simulink model "mbr_2048ch_983mhz_real2"  (R2021a) (continued)
== system mbr_2048ch_983mhz_real2 == (continued)
  synth3_2 : Subsystem  Tag=xps:bram  AncestorBlock=xps_library/Memory/shared_bram  mask{arith_type=Unsigned, addr_width=9, data_width=64, reg_prim_output=on, reg_core_output=on, optimization=Minimum_Area, data_bin_pt=0, init_vals=[0:2^9-1], sample_rate=1}
      mask prompts: arith_type "Output Data Type"; addr_width "Address width"; data_width "Data Width"; reg_prim_output "Register Primitive Output"; reg_core_output "Register Core Output"; data_bin_pt "Data Binary Point"; init_vals "Initial values (simulation only)"; sample_rate "Sample rate"
      mask init:
      | shared_bram_mask;
      mask TypeOptions arith_type: Unsigned | Signed  (2's comp)
      mask TypeOptions data_width: 8 | 16 | 32 | 64 | 128
      mask TypeOptions optimization: Minimum_Area | Low_Power
  synth3_3 : Subsystem  Tag=xps:bram  AncestorBlock=xps_library/Memory/shared_bram  mask{arith_type=Unsigned, addr_width=9, data_width=64, reg_prim_output=on, reg_core_output=on, optimization=Minimum_Area, data_bin_pt=0, init_vals=[0:2^9-1], sample_rate=1}
      mask prompts: arith_type "Output Data Type"; addr_width "Address width"; data_width "Data Width"; reg_prim_output "Register Primitive Output"; reg_core_output "Register Core Output"; data_bin_pt "Data Binary Point"; init_vals "Initial values (simulation only)"; sample_rate "Sample rate"
      mask init:
      | shared_bram_mask;
      mask TypeOptions arith_type: Unsigned | Signed  (2's comp)
      mask TypeOptions data_width: 8 | 16 | 32 | 64 | 128
      mask TypeOptions optimization: Minimum_Area | Low_Power
  wires:
  Constant/1 -> adc_snapshot_d/3(trig), adc_snapshot_d/2(we)
  pipeline11/1(q) -> spect_cnt/1
  a2_1/1(data_out) -> Terminator14/1
  power4/1 -> Delay8/1
  fft_wideband_real/2(out00) -> Delay15/1
  Delay10/1 -> bram_mult2_3/1(In1), bram_mult2_2/1(In1), bram_mult2_1/1(In1), bram_mult2_0/1(In1), pipeline162/1(d), Delay34/1
  rfdc/1(m00_axis_tdata) -> munge/1(din), Reinterpret9/1
  fft_wideband_real/6(of) -> Terminator/1
  simple_bram_vacc/1(dout) -> Reinterpret4/1
  a2_0/1(data_out) -> Terminator13/1
  Reinterpret4/1 -> pipeline24/1(d)
  Constant19/1 -> cnt_rst/1(sim_1)
  a2_3/1(data_out) -> Terminator3/1
  Delay15/1 -> Delay5/1
  fft_wideband_real/1(sync_out) -> Delay14/1
  Delay6/1 -> Delay7/1
  fft_wideband_real/3(out01) -> Delay16/1
  fft_wideband_real/4(out02) -> Delay2/1
  Delay14/1 -> Delay10/1
  Delay23/1 -> pipeline3/1(d)
  power3/1 -> Delay4/1
  power2/1 -> Delay26/1
  Constant16/1 -> acc_len/1(sim_1)
  simple_bram_vacc/2(valid) -> pipeline25/1(d), spect_cnt/2
  simple_bram_vacc2/1(dout) -> Reinterpret1/1
  Delay24/1 -> acc_cntrl/2(sync)
  sync_gen/1(sync_out) -> Delay23/1
  pipeline8/1(q) -> [acc_len]
  Delay16/1 -> Delay17/1
  a2_2/1(data_out) -> Terminator2/1
  pipeline9/1(q) -> [cnt_rst]
  Delay34/1 -> Delay24/1
  [cnt_rst] -> acc_cntrl/3(rst)
  Constant2/1 -> fft_wideband_real/2(shift)
  simple_bram_vacc3/1(dout) -> Reinterpret2/1
  pipeline3/1(q) -> fft_wideband_real/1(sync)
  cnt_rst/1(in_reg) -> pipeline9/1(d)
  Delay2/1 -> Delay3/1
  munge/1(dout) -> bus_expand/1(bus_in)
  power1/1 -> Delay25/1
  [acc_len] -> acc_cntrl/1(acc_len)
  acc_len/1(in_reg) -> pipeline8/1(d)
  simple_bram_vacc1/1(dout) -> Reinterpret5/1
  fft_wideband_real/5(out03) -> Delay6/1
  adc_snapshot_d/1(arm_out) -> Terminator4/1
  Reinterpret9/1 -> adc_snapshot_d/1(in_d)
  Reinterpret5/1 -> pipeline16/1(d)
  simple_bram_vacc1/2(valid) -> pipeline17/1(d)
  Reinterpret1/1 -> pipeline18/1(d)
  Reinterpret2/1 -> pipeline21/1(d)
  simple_bram_vacc3/2(valid) -> pipeline23/1(d)
  simple_bram_vacc2/2(valid) -> pipeline19/1(d)
  pipeline5/1(q) -> simple_bram_vacc/1(new_acc)
  pipeline6/1(q) -> simple_bram_vacc/2(din)
  pipeline12/1(q) -> simple_bram_vacc1/1(new_acc)
  pipeline13/1(q) -> simple_bram_vacc1/2(din)
  pipeline22/1(q) -> simple_bram_vacc2/1(new_acc)
  pipeline27/1(q) -> simple_bram_vacc2/2(din)
  pipeline14/1(q) -> simple_bram_vacc3/1(new_acc)
  pipeline15/1(q) -> simple_bram_vacc3/2(din)
  acc_cntrl/1(new_acc) -> pipeline11/1(d), pipeline82/1(d), pipeline90/1(d), pipeline80/1(d), pipeline100/1(d), pipeline59/1(d), pipeline67/1(d), pipeline57/1(d), pipeline77/1(d), pipeline14/1(d), pipeline22/1(d), pipeline12/1(d), pipeline5/1(d)
  pipeline29/1(q) -> pipeline6/1(d)
  pipeline30/1(q) -> pipeline13/1(d)
  pipeline31/1(q) -> pipeline27/1(d)
  pipeline32/1(q) -> pipeline15/1(d)
  Delay25/1 -> pipeline29/1(d)
  Delay26/1 -> pipeline30/1(d)
  Delay4/1 -> pipeline31/1(d)
  Delay8/1 -> pipeline32/1(d)
  Delay33/1 -> pipeline51/1(d)
  Delay29/1 -> pipeline50/1(d)
  Delay22/1 -> pipeline49/1(d)
  Delay21/1 -> pipeline47/1(d)
  pipeline51/1(q) -> pipeline34/1(d)
  pipeline50/1(q) -> pipeline46/1(d)
  pipeline49/1(q) -> pipeline28/1(d)
  pipeline47/1(q) -> pipeline53/1(d)
  acc_cntrl1/1(new_acc) -> pipeline52/1(d), pipeline10/1(d), pipeline41/1(d), pipeline33/1(d), pipeline4/1(d)
  pipeline34/1(q) -> simple_bram_vacc7/2(din)
  pipeline33/1(q) -> simple_bram_vacc7/1(new_acc)
  pipeline46/1(q) -> simple_bram_vacc6/2(din)
  pipeline41/1(q) -> simple_bram_vacc6/1(new_acc)
  pipeline28/1(q) -> simple_bram_vacc5/2(din)
  pipeline10/1(q) -> simple_bram_vacc5/1(new_acc)
  pipeline53/1(q) -> simple_bram_vacc4/2(din)
  pipeline52/1(q) -> simple_bram_vacc4/1(new_acc)
  simple_bram_vacc6/2(valid) -> pipeline38/1(d)
  simple_bram_vacc7/2(valid) -> pipeline42/1(d)
  Reinterpret6/1 -> pipeline40/1(d)
  Reinterpret3/1 -> pipeline37/1(d)
  simple_bram_vacc5/2(valid) -> pipeline36/1(d)
  Reinterpret8/1 -> pipeline35/1(d)
  Reinterpret10/1 -> adc_snapshot_c/1(in_d)
  adc_snapshot_c/1(arm_out) -> Terminator9/1
  fft_wideband_real1/5(out03) -> Delay31/1
  simple_bram_vacc5/1(dout) -> Reinterpret8/1
  Delay27/1 -> power7/1, cmult2/1(a), pipeline155/1(d), bram_mult2_2/2(d)
  power5/1 -> Delay21/1
  munge1/1(dout) -> bus_expand1/1(bus_in)
  Delay18/1 -> Delay27/1
  pipeline48/1(q) -> fft_wideband_real1/1(sync)
  simple_bram_vacc7/1(dout) -> Reinterpret6/1
  Constant3/1 -> fft_wideband_real1/2(shift)
  [cnt_rst] -> acc_cntrl1/3(rst)
  Delay28/1 -> Delay20/1
  b2_2/1(data_out) -> Terminator7/1
  Delay12/1 -> Delay13/1
  sync_gen1/1(sync_out) -> Delay19/1
  Delay20/1 -> acc_cntrl1/2(sync)
  simple_bram_vacc6/1(dout) -> Reinterpret3/1
  simple_bram_vacc4/2(valid) -> spect_cnt1/2, pipeline44/1(d)
  power6/1 -> Delay22/1
  power7/1 -> Delay29/1
  Delay19/1 -> pipeline48/1(d)
  Delay9/1 -> Delay1/1
  fft_wideband_real1/4(out02) -> Delay18/1
  fft_wideband_real1/3(out01) -> Delay12/1
  Delay31/1 -> Delay32/1
  fft_wideband_real1/1(sync_out) -> Delay9/1
  Delay11/1 -> Delay30/1
  Delay13/1 -> power6/1, cmult1/1(a), pipeline154/1(d), bram_mult2_1/2(d)
  b2_3/1(data_out) -> Terminator8/1
  Reinterpret7/1 -> pipeline43/1(d)
  b2_0/1(data_out) -> Terminator5/1
  spect_cnt1/1 -> pipeline45/1(d), pipeline39/1(d), pipeline54/1(d), pipeline2/1(d)
  simple_bram_vacc4/1(dout) -> Reinterpret7/1
  Delay32/1 -> power8/1, cmult3/1(a), pipeline156/1(d), bram_mult2_3/2(d)
  Delay30/1 -> cmult/1(a), pipeline153/1(d), bram_mult2_0/2(d), power5/1
  fft_wideband_real1/6(of) -> Terminator1/1
  Delay1/1 -> pipeline164/1(d), bram_mult3_0/1(In1), bram_mult3_1/1(In1), bram_mult3_2/1(In1), bram_mult3_3/1(In1), Delay28/1
  fft_wideband_real1/2(out00) -> Delay11/1
  power8/1 -> Delay33/1
  b2_1/1(data_out) -> Terminator6/1
  pipeline4/1(q) -> spect_cnt1/1
  Constant1/1 -> adc_snapshot_c/2(we), adc_snapshot_c/3(trig)
  rfdc/2(m02_axis_tdata) -> Reinterpret10/1, munge1/1(din)
  [acc_len] -> acc_cntrl1/1(acc_len)
  rfdc/3(m10_axis_tdata) -> Terminator10/1
  rfdc/4(m12_axis_tdata) -> Terminator11/1
  rfdc/8(m32_axis_tdata) -> Terminator17/1
  rfdc/7(m30_axis_tdata) -> Terminator16/1
  Band-Limited White Noise/1 -> rfdc/8(m32_axis_tdata_sim), rfdc/7(m30_axis_tdata_sim), rfdc/6(m22_axis_tdata_sim), rfdc/5(m20_axis_tdata_sim), rfdc/4(m12_axis_tdata_sim), rfdc/3(m10_axis_tdata_sim), rfdc/2(m02_axis_tdata_sim), rfdc/1(m00_axis_tdata_sim)
  Delay40/1 -> pipeline76/1(d)
  Delay39/1 -> pipeline75/1(d)
  Delay37/1 -> pipeline74/1(d)
  Delay36/1 -> pipeline73/1(d)
  pipeline76/1(q) -> pipeline60/1(d)
  pipeline75/1(q) -> pipeline72/1(d)
  pipeline74/1(q) -> pipeline58/1(d)
  pipeline73/1(q) -> pipeline78/1(d)
  pipeline60/1(q) -> simple_bram_vacc11/2(din)
  pipeline59/1(q) -> simple_bram_vacc11/1(new_acc)
  pipeline72/1(q) -> simple_bram_vacc10/2(din)
  pipeline67/1(q) -> simple_bram_vacc10/1(new_acc)
  pipeline58/1(q) -> simple_bram_vacc9/2(din)
  pipeline57/1(q) -> simple_bram_vacc9/1(new_acc)
  pipeline78/1(q) -> simple_bram_vacc8/2(din)
  pipeline77/1(q) -> simple_bram_vacc8/1(new_acc)
  simple_bram_vacc10/2(valid) -> pipeline64/1(d)
  simple_bram_vacc11/2(valid) -> pipeline68/1(d)
  Reinterpret12/1 -> pipeline66/1(d)
  Reinterpret11/1 -> pipeline63/1(d)
  simple_bram_vacc9/2(valid) -> pipeline62/1(d)
  Reinterpret14/1 -> pipeline61/1(d)
  simple_bram_vacc9/1(dout) -> Reinterpret14/1
  c_to_ri/1 -> Delay36/1
  simple_bram_vacc11/1(dout) -> Reinterpret12/1
  ab_re1/1(data_out) -> Terminator20/1
  simple_bram_vacc10/1(dout) -> Reinterpret11/1
  simple_bram_vacc8/2(valid) -> pipeline70/1(d)
  c_to_ri/2 -> Delay37/1
  c_to_ri1/1 -> Delay39/1
  ab_im1/1(data_out) -> Terminator21/1
  Reinterpret13/1 -> pipeline69/1(d)
  ab_re0/1(data_out) -> Terminator18/1
  spect_cnt/1 -> pipeline1/1(d), pipeline7/1(d), pipeline20/1(d), pipeline56/1(d), pipeline94/1(d), pipeline88/1(d), pipeline102/1(d), pipeline71/1(d), pipeline65/1(d), pipeline79/1(d), pipeline55/1(d), pipeline26/1(d)
  simple_bram_vacc8/1(dout) -> Reinterpret13/1
  c_to_ri1/2 -> Delay40/1
  ab_im0/1(data_out) -> Terminator19/1
  ab_im2/1(data_out) -> Terminator23/1
  c_to_ri3/2 -> Delay42/1
  simple_bram_vacc14/1(dout) -> Reinterpret17/1
  ab_re2/1(data_out) -> Terminator22/1
  Reinterpret17/1 -> pipeline92/1(d)
  ab_im3/1(data_out) -> Terminator25/1
  c_to_ri3/1 -> Delay41/1
  c_to_ri2/2 -> Delay38/1
  simple_bram_vacc14/2(valid) -> pipeline93/1(d)
  simple_bram_vacc12/1(dout) -> Reinterpret15/1
  ab_re3/1(data_out) -> Terminator24/1
  simple_bram_vacc13/1(dout) -> Reinterpret16/1
  c_to_ri2/1 -> Delay35/1
  simple_bram_vacc15/1(dout) -> Reinterpret18/1
  Reinterpret18/1 -> pipeline84/1(d)
  simple_bram_vacc15/2(valid) -> pipeline85/1(d)
  Reinterpret15/1 -> pipeline86/1(d)
  Reinterpret16/1 -> pipeline89/1(d)
  simple_bram_vacc13/2(valid) -> pipeline91/1(d)
  simple_bram_vacc12/2(valid) -> pipeline87/1(d)
  pipeline100/1(q) -> simple_bram_vacc14/1(new_acc)
  pipeline101/1(q) -> simple_bram_vacc14/2(din)
  pipeline80/1(q) -> simple_bram_vacc15/1(new_acc)
  pipeline81/1(q) -> simple_bram_vacc15/2(din)
  pipeline90/1(q) -> simple_bram_vacc12/1(new_acc)
  pipeline95/1(q) -> simple_bram_vacc12/2(din)
  pipeline82/1(q) -> simple_bram_vacc13/1(new_acc)
  pipeline83/1(q) -> simple_bram_vacc13/2(din)
  pipeline96/1(q) -> pipeline101/1(d)
  pipeline97/1(q) -> pipeline81/1(d)
  pipeline98/1(q) -> pipeline95/1(d)
  pipeline99/1(q) -> pipeline83/1(d)
  Delay35/1 -> pipeline96/1(d)
  Delay38/1 -> pipeline97/1(d)
  Delay41/1 -> pipeline98/1(d)
  Delay42/1 -> pipeline99/1(d)
  cmult/1(ab) -> c_to_ri/1
  cmult1/1(ab) -> c_to_ri1/1
  cmult3/1(ab) -> c_to_ri3/1
  cmult2/1(ab) -> c_to_ri2/1
  [acc_len] -> acc_cntrl2/1(acc_len)
  pipeline113/1(q) -> spect_cnt2/1
  synth1_1/1(data_out) -> Terminator27/1
  power12/1 -> Delay48/1
  simple_bram_vacc16/1(dout) -> Reinterpret21/1
  spect_cnt2/1 -> pipeline104/1(d), pipeline127/1(d), pipeline112/1(d), pipeline119/1(d)
  synth1_0/1(data_out) -> Terminator26/1
  Reinterpret21/1 -> pipeline117/1(d)
  synth1_3/1(data_out) -> Terminator29/1
  power11/1 -> Delay47/1
  power10/1 -> Delay45/1
  simple_bram_vacc16/2(valid) -> pipeline118/1(d), spect_cnt2/2
  simple_bram_vacc18/1(dout) -> Reinterpret19/1
  Delay43/1 -> acc_cntrl2/2(sync)
  synth1_2/1(data_out) -> Terminator28/1
  Delay46/1 -> Delay43/1
  [cnt_rst] -> acc_cntrl2/3(rst)
  simple_bram_vacc19/1(dout) -> Reinterpret20/1
  power9/1 -> Delay44/1
  simple_bram_vacc17/1(dout) -> Reinterpret22/1
  Reinterpret22/1 -> pipeline108/1(d)
  simple_bram_vacc17/2(valid) -> pipeline109/1(d)
  Reinterpret19/1 -> pipeline110/1(d)
  Reinterpret20/1 -> pipeline114/1(d)
  simple_bram_vacc19/2(valid) -> pipeline116/1(d)
  simple_bram_vacc18/2(valid) -> pipeline111/1(d)
  pipeline125/1(q) -> simple_bram_vacc16/1(new_acc)
  pipeline126/1(q) -> simple_bram_vacc16/2(din)
  pipeline103/1(q) -> simple_bram_vacc17/1(new_acc)
  pipeline105/1(q) -> simple_bram_vacc17/2(din)
  pipeline115/1(q) -> simple_bram_vacc18/1(new_acc)
  pipeline120/1(q) -> simple_bram_vacc18/2(din)
  pipeline106/1(q) -> simple_bram_vacc19/1(new_acc)
  pipeline107/1(q) -> simple_bram_vacc19/2(din)
  acc_cntrl2/1(new_acc) -> pipeline113/1(d), pipeline106/1(d), pipeline115/1(d), pipeline103/1(d), pipeline125/1(d)
  pipeline121/1(q) -> pipeline126/1(d)
  pipeline122/1(q) -> pipeline105/1(d)
  pipeline123/1(q) -> pipeline120/1(d)
  pipeline124/1(q) -> pipeline107/1(d)
  Delay44/1 -> pipeline121/1(d)
  Delay45/1 -> pipeline122/1(d)
  Delay47/1 -> pipeline123/1(d)
  Delay48/1 -> pipeline124/1(d)
  Delay54/1 -> pipeline149/1(d)
  Delay53/1 -> pipeline148/1(d)
  Delay51/1 -> pipeline147/1(d)
  Delay50/1 -> pipeline146/1(d)
  pipeline149/1(q) -> pipeline132/1(d)
  pipeline148/1(q) -> pipeline145/1(d)
  pipeline147/1(q) -> pipeline130/1(d)
  pipeline146/1(q) -> pipeline151/1(d)
  acc_cntrl3/1(new_acc) -> pipeline150/1(d), pipeline128/1(d), pipeline140/1(d), pipeline131/1(d), pipeline138/1(d)
  pipeline132/1(q) -> simple_bram_vacc23/2(din)
  pipeline131/1(q) -> simple_bram_vacc23/1(new_acc)
  pipeline145/1(q) -> simple_bram_vacc22/2(din)
  pipeline140/1(q) -> simple_bram_vacc22/1(new_acc)
  pipeline130/1(q) -> simple_bram_vacc21/2(din)
  pipeline128/1(q) -> simple_bram_vacc21/1(new_acc)
  pipeline151/1(q) -> simple_bram_vacc20/2(din)
  pipeline150/1(q) -> simple_bram_vacc20/1(new_acc)
  simple_bram_vacc22/2(valid) -> pipeline136/1(d)
  simple_bram_vacc23/2(valid) -> pipeline141/1(d)
  Reinterpret24/1 -> pipeline139/1(d)
  Reinterpret23/1 -> pipeline135/1(d)
  simple_bram_vacc21/2(valid) -> pipeline134/1(d)
  Reinterpret26/1 -> pipeline133/1(d)
  simple_bram_vacc21/1(dout) -> Reinterpret26/1
  power16/1 -> Delay50/1
  simple_bram_vacc23/1(dout) -> Reinterpret24/1
  [cnt_rst] -> acc_cntrl3/3(rst)
  Delay52/1 -> Delay49/1
  synth0_2/1(data_out) -> Terminator32/1
  Delay49/1 -> acc_cntrl3/2(sync)
  simple_bram_vacc22/1(dout) -> Reinterpret23/1
  simple_bram_vacc20/2(valid) -> spect_cnt3/2, pipeline143/1(d)
  power13/1 -> Delay51/1
  power14/1 -> Delay53/1
  synth0_3/1(data_out) -> Terminator33/1
  Reinterpret25/1 -> pipeline142/1(d)
  synth0_0/1(data_out) -> Terminator30/1
  spect_cnt3/1 -> pipeline144/1(d), pipeline137/1(d), pipeline152/1(d), pipeline129/1(d)
  simple_bram_vacc20/1(dout) -> Reinterpret25/1
  power15/1 -> Delay54/1
  synth0_1/1(data_out) -> Terminator31/1
  pipeline138/1(q) -> spect_cnt3/1
  [acc_len] -> acc_cntrl3/1(acc_len)
  comp_add4/1(a+b) -> power16/1
  comp_add5/1(a+b) -> power13/1
  comp_add6/1(a+b) -> power14/1
  comp_add7/1(a+b) -> power15/1
  bram_mult3_0/1(c) -> comp_add4/2(b)
  pipeline153/1(q) -> comp_add4/1(a)
  bram_mult3_1/1(c) -> comp_add5/2(b)
  pipeline154/1(q) -> comp_add5/1(a)
  pipeline156/1(q) -> comp_add7/1(a)
  bram_mult3_3/1(c) -> comp_add7/2(b)
  pipeline155/1(q) -> comp_add6/1(a)
  bram_mult3_2/1(c) -> comp_add6/2(b)
  bram_mult2_2/1(c) -> comp_add2/2(b)
  pipeline159/1(q) -> comp_add2/1(a)
  bram_mult2_3/1(c) -> comp_add3/2(b)
  pipeline160/1(q) -> comp_add3/1(a)
  pipeline158/1(q) -> comp_add1/1(a)
  bram_mult2_1/1(c) -> comp_add1/2(b)
  pipeline157/1(q) -> comp_add/1(a)
  bram_mult2_0/1(c) -> comp_add/2(b)
  comp_add3/1(a+b) -> Delay112/1, power12/1
  comp_add2/1(a+b) -> Delay111/1, power11/1
  comp_add1/1(a+b) -> Delay110/1, power10/1
  comp_add/1(a+b) -> Delay109/1, power9/1
  pipeline161/1(q) -> Delay46/1, Delay113/1
  pipeline162/1(q) -> pipeline161/1(d)
  pipeline164/1(q) -> pipeline163/1(d)
  pipeline163/1(q) -> Delay52/1
  Delay5/1 -> cmult/2(b), bram_mult3_0/2(d), pipeline157/1(d), power1/1
  Delay17/1 -> power2/1, cmult1/2(b), bram_mult3_1/2(d), pipeline158/1(d)
  Delay3/1 -> power3/1, cmult2/2(b), bram_mult3_2/2(d), pipeline159/1(d)
  Delay7/1 -> power4/1, cmult3/2(b), bram_mult3_3/2(d), pipeline160/1(d)
  Delay106/1 -> power27/1, bram_mult1_3/2(d), pipeline228/1(d), cmult7/2(b)
  Delay77/1 -> bram_mult1_2/2(d), pipeline227/1(d), power26/1, cmult6/2(b)
  Delay63/1 -> cmult5/2(b), power25/1, bram_mult1_1/2(d), pipeline226/1(d)
  Delay99/1 -> bram_mult1_0/2(d), pipeline225/1(d), cmult4/2(b), power17/1
  pipeline236/1(q) -> Delay102/1
  pipeline237/1(q) -> pipeline236/1(d)
  pipeline235/1(q) -> pipeline234/1(d)
  pipeline234/1(q) -> inverter4/1(sync), inverter1/1(sync), inverter2/1(sync), inverter3/1(sync), Delay95/1
  comp_add8/1(a+b) -> inverter3/2(din), power32/1
  comp_add9/1(a+b) -> inverter2/2(din), power18/1
  comp_add10/1(a+b) -> inverter1/2(din), power19/1
  comp_add11/1(a+b) -> inverter4/2(din), power20/1
  bram_mult1_0/1(c) -> comp_add8/2(b)
  pipeline229/1(q) -> comp_add8/1(a)
  bram_mult1_1/1(c) -> comp_add9/2(b)
  pipeline230/1(q) -> comp_add9/1(a)
  pipeline233/1(q) -> comp_add11/1(a)
  bram_mult1_3/1(c) -> comp_add11/2(b)
  pipeline231/1(q) -> comp_add10/1(a)
  bram_mult1_2/1(c) -> comp_add10/2(b)
  bram_mult0_2/1(c) -> comp_add14/2(b)
  pipeline227/1(q) -> comp_add14/1(a)
  bram_mult0_3/1(c) -> comp_add15/2(b)
  pipeline228/1(q) -> comp_add15/1(a)
  pipeline226/1(q) -> comp_add13/1(a)
  bram_mult0_1/1(c) -> comp_add13/2(b)
  pipeline225/1(q) -> comp_add12/1(a)
  bram_mult0_0/1(c) -> comp_add12/2(b)
  comp_add15/1(a+b) -> power23/1
  comp_add14/1(a+b) -> power22/1
  comp_add13/1(a+b) -> power21/1
  comp_add12/1(a+b) -> power24/1
  [acc_len] -> acc_cntrl7/1(acc_len)
  pipeline208/1(q) -> spect_cnt7/1
  synth3_1/1(data_out) -> Terminator53/1
  power23/1 -> Delay104/1
  simple_bram_vacc37/1(dout) -> Reinterpret44/1
  spect_cnt7/1 -> pipeline198/1(d), pipeline224/1(d), pipeline207/1(d), pipeline215/1(d)
  synth3_0/1(data_out) -> Terminator52/1
  Reinterpret44/1 -> pipeline213/1(d)
  synth3_3/1(data_out) -> Terminator55/1
  power22/1 -> Delay103/1
  power21/1 -> Delay101/1
  simple_bram_vacc37/2(valid) -> pipeline214/1(d), spect_cnt7/2
  simple_bram_vacc39/1(dout) -> Reinterpret42/1
  Delay98/1 -> acc_cntrl7/2(sync)
  synth3_2/1(data_out) -> Terminator54/1
  Delay102/1 -> Delay98/1
  [cnt_rst] -> acc_cntrl7/3(rst)
  simple_bram_vacc40/1(dout) -> Reinterpret43/1
  power24/1 -> Delay100/1
  simple_bram_vacc38/1(dout) -> Reinterpret45/1
  Reinterpret45/1 -> pipeline203/1(d)
  simple_bram_vacc38/2(valid) -> pipeline204/1(d)
  Reinterpret42/1 -> pipeline205/1(d)
  Reinterpret43/1 -> pipeline209/1(d)
  simple_bram_vacc40/2(valid) -> pipeline212/1(d)
  simple_bram_vacc39/2(valid) -> pipeline206/1(d)
  pipeline222/1(q) -> simple_bram_vacc37/1(new_acc)
  pipeline223/1(q) -> simple_bram_vacc37/2(din)
  pipeline197/1(q) -> simple_bram_vacc38/1(new_acc)
  pipeline200/1(q) -> simple_bram_vacc38/2(din)
  pipeline211/1(q) -> simple_bram_vacc39/1(new_acc)
  pipeline216/1(q) -> simple_bram_vacc39/2(din)
  pipeline201/1(q) -> simple_bram_vacc40/1(new_acc)
  pipeline202/1(q) -> simple_bram_vacc40/2(din)
  acc_cntrl7/1(new_acc) -> pipeline208/1(d), pipeline201/1(d), pipeline211/1(d), pipeline197/1(d), pipeline222/1(d)
  pipeline217/1(q) -> pipeline223/1(d)
  pipeline218/1(q) -> pipeline200/1(d)
  pipeline219/1(q) -> pipeline216/1(d)
  pipeline220/1(q) -> pipeline202/1(d)
  Delay100/1 -> pipeline217/1(d)
  Delay101/1 -> pipeline218/1(d)
  Delay103/1 -> pipeline219/1(d)
  Delay104/1 -> pipeline220/1(d)
  Delay97/1 -> pipeline193/1(d)
  Delay96/1 -> pipeline192/1(d)
  Delay94/1 -> pipeline191/1(d)
  Delay93/1 -> pipeline190/1(d)
  pipeline193/1(q) -> pipeline174/1(d)
  pipeline192/1(q) -> pipeline189/1(d)
  pipeline191/1(q) -> pipeline172/1(d)
  pipeline190/1(q) -> pipeline195/1(d)
  acc_cntrl6/1(new_acc) -> pipeline194/1(d), pipeline170/1(d), pipeline183/1(d), pipeline173/1(d), pipeline181/1(d)
  pipeline174/1(q) -> simple_bram_vacc35/2(din)
  pipeline173/1(q) -> simple_bram_vacc35/1(new_acc)
  pipeline189/1(q) -> simple_bram_vacc34/2(din)
  pipeline183/1(q) -> simple_bram_vacc34/1(new_acc)
  pipeline172/1(q) -> simple_bram_vacc33/2(din)
  pipeline170/1(q) -> simple_bram_vacc33/1(new_acc)
  pipeline195/1(q) -> simple_bram_vacc32/2(din)
  pipeline194/1(q) -> simple_bram_vacc32/1(new_acc)
  simple_bram_vacc34/2(valid) -> pipeline179/1(d)
  simple_bram_vacc35/2(valid) -> pipeline184/1(d)
  Reinterpret39/1 -> pipeline182/1(d)
  Reinterpret37/1 -> pipeline178/1(d)
  simple_bram_vacc33/2(valid) -> pipeline176/1(d)
  Reinterpret41/1 -> pipeline175/1(d)
  simple_bram_vacc33/1(dout) -> Reinterpret41/1
  power32/1 -> Delay93/1
  simple_bram_vacc35/1(dout) -> Reinterpret39/1
  [cnt_rst] -> acc_cntrl6/3(rst)
  Delay95/1 -> Delay92/1
  synth2_2/1(data_out) -> Terminator49/1
  Delay92/1 -> acc_cntrl6/2(sync)
  simple_bram_vacc34/1(dout) -> Reinterpret37/1
  simple_bram_vacc32/2(valid) -> spect_cnt6/2, pipeline186/1(d)
  power18/1 -> Delay94/1
  power19/1 -> Delay96/1
  synth2_3/1(data_out) -> Terminator50/1
  Reinterpret40/1 -> pipeline185/1(d)
  synth2_0/1(data_out) -> Terminator47/1
  spect_cnt6/1 -> pipeline187/1(d), pipeline180/1(d), pipeline196/1(d), pipeline171/1(d)
  simple_bram_vacc32/1(dout) -> Reinterpret40/1
  power20/1 -> Delay97/1
  synth2_1/1(data_out) -> Terminator48/1
  pipeline181/1(q) -> spect_cnt6/1
  [acc_len] -> acc_cntrl6/1(acc_len)
  cmult6/1(ab) -> c_to_ri6/1
  cmult7/1(ab) -> c_to_ri7/1
  cmult5/1(ab) -> c_to_ri5/1
  cmult4/1(ab) -> c_to_ri4/1
  Delay91/1 -> pipeline326/1(d)
  Delay90/1 -> pipeline325/1(d)
  Delay86/1 -> pipeline324/1(d)
  Delay83/1 -> pipeline323/1(d)
  pipeline326/1(q) -> pipeline310/1(d)
  pipeline325/1(q) -> pipeline322/1(d)
  pipeline324/1(q) -> pipeline308/1(d)
  pipeline323/1(q) -> pipeline168/1(d)
  pipeline310/1(q) -> simple_bram_vacc29/2(din)
  pipeline309/1(q) -> simple_bram_vacc29/1(new_acc)
  pipeline322/1(q) -> simple_bram_vacc28/2(din)
  pipeline317/1(q) -> simple_bram_vacc28/1(new_acc)
  pipeline308/1(q) -> simple_bram_vacc31/2(din)
  pipeline307/1(q) -> simple_bram_vacc31/1(new_acc)
  pipeline168/1(q) -> simple_bram_vacc30/2(din)
  pipeline167/1(q) -> simple_bram_vacc30/1(new_acc)
  simple_bram_vacc28/2(valid) -> pipeline314/1(d)
  simple_bram_vacc29/2(valid) -> pipeline318/1(d)
  Reinterpret34/1 -> pipeline316/1(d)
  Reinterpret33/1 -> pipeline313/1(d)
  simple_bram_vacc31/2(valid) -> pipeline312/1(d)
  Reinterpret36/1 -> pipeline311/1(d)
  simple_bram_vacc31/1(dout) -> Reinterpret36/1
  c_to_ri6/1 -> Delay83/1
  simple_bram_vacc29/1(dout) -> Reinterpret34/1
  cd_re3/1(data_out) -> Terminator45/1
  simple_bram_vacc28/1(dout) -> Reinterpret33/1
  simple_bram_vacc30/2(valid) -> pipeline320/1(d)
  c_to_ri6/2 -> Delay86/1
  c_to_ri7/1 -> Delay90/1
  cd_im3/1(data_out) -> Terminator46/1
  Reinterpret35/1 -> pipeline319/1(d)
  cd_re2/1(data_out) -> Terminator43/1
  simple_bram_vacc30/1(dout) -> Reinterpret35/1
  c_to_ri7/2 -> Delay91/1
  cd_im2/1(data_out) -> Terminator44/1
  cd_im0/1(data_out) -> Terminator39/1
  c_to_ri5/2 -> Delay89/1
  simple_bram_vacc46/1(dout) -> Reinterpret31/1
  spect_cnt4/1 -> pipeline248/1(d), pipeline280/1(d), pipeline306/1(d), pipeline291/1(d), pipeline298/1(d), pipeline169/1(d), pipeline315/1(d), pipeline321/1(d), pipeline281/1(d), pipeline242/1(d), pipeline296/1(d), pipeline165/1(d)
  cd_re0/1(data_out) -> Terminator38/1
  Reinterpret31/1 -> pipeline295/1(d)
  cd_im1/1(data_out) -> Terminator42/1
  c_to_ri5/1 -> Delay87/1
  c_to_ri4/2 -> Delay85/1
  simple_bram_vacc46/2(valid) -> pipeline297/1(d)
  simple_bram_vacc26/1(dout) -> Reinterpret29/1
  cd_re1/1(data_out) -> Terminator41/1
  simple_bram_vacc27/1(dout) -> Reinterpret30/1
  c_to_ri4/1 -> Delay84/1
  simple_bram_vacc47/1(dout) -> Reinterpret32/1
  Reinterpret32/1 -> pipeline287/1(d)
  simple_bram_vacc47/2(valid) -> pipeline288/1(d)
  Reinterpret29/1 -> pipeline289/1(d)
  Reinterpret30/1 -> pipeline292/1(d)
  simple_bram_vacc27/2(valid) -> pipeline294/1(d)
  simple_bram_vacc26/2(valid) -> pipeline290/1(d)
  pipeline304/1(q) -> simple_bram_vacc46/1(new_acc)
  pipeline305/1(q) -> simple_bram_vacc46/2(din)
  pipeline282/1(q) -> simple_bram_vacc47/1(new_acc)
  pipeline283/1(q) -> simple_bram_vacc47/2(din)
  pipeline293/1(q) -> simple_bram_vacc26/1(new_acc)
  pipeline299/1(q) -> simple_bram_vacc26/2(din)
  pipeline284/1(q) -> simple_bram_vacc27/1(new_acc)
  pipeline286/1(q) -> simple_bram_vacc27/2(din)
  pipeline300/1(q) -> pipeline305/1(d)
  pipeline301/1(q) -> pipeline283/1(d)
  pipeline302/1(q) -> pipeline299/1(d)
  pipeline303/1(q) -> pipeline286/1(d)
  Delay84/1 -> pipeline300/1(d)
  Delay85/1 -> pipeline301/1(d)
  Delay87/1 -> pipeline302/1(d)
  Delay89/1 -> pipeline303/1(d)
  [acc_len] -> acc_cntrl5/1(acc_len)
  rfdc/6(m22_axis_tdata) -> Reinterpret28/1, munge3/1(din)
  Constant5/1 -> adc_snapshot_a/3(trig), adc_snapshot_a/2(we)
  pipeline263/1(q) -> spect_cnt5/1
  d2_1/1(data_out) -> Terminator58/1
  power31/1 -> Delay81/1
  fft_wideband_real3/2(out00) -> Delay57/1
  Delay55/1 -> bram_mult1_0/1(In1), bram_mult1_3/1(In1), bram_mult1_2/1(In1), bram_mult1_1/1(In1), pipeline235/1(d), Delay75/1
  fft_wideband_real3/6(of) -> Terminator35/1
  Delay78/1 -> power28/1, cmult4/1(a), bram_mult0_0/2(d), pipeline229/1(d)
  Delay80/1 -> power31/1, cmult7/1(a), bram_mult0_3/2(d), pipeline233/1(d)
  simple_bram_vacc42/1(dout) -> Reinterpret50/1
  spect_cnt5/1 -> pipeline241/1(d), pipeline279/1(d), pipeline262/1(d), pipeline269/1(d)
  d2_0/1(data_out) -> Terminator57/1
  Reinterpret50/1 -> pipeline267/1(d)
  d2_3/1(data_out) -> Terminator60/1
  Delay57/1 -> Delay78/1
  fft_wideband_real3/1(sync_out) -> Delay108/1
  Delay79/1 -> Delay80/1
  fft_wideband_real3/3(out01) -> Delay58/1
  fft_wideband_real3/4(out02) -> Delay64/1
  Delay108/1 -> Delay55/1
  Delay65/1 -> pipeline272/1(d)
  power30/1 -> Delay76/1
  power29/1 -> Delay69/1
  simple_bram_vacc42/2(valid) -> pipeline268/1(d), spect_cnt5/2
  simple_bram_vacc44/1(dout) -> Reinterpret46/1
  Delay67/1 -> acc_cntrl5/2(sync)
  sync_gen3/1(sync_out) -> Delay65/1
  Delay58/1 -> Delay59/1
  d2_2/1(data_out) -> Terminator59/1
  Delay75/1 -> Delay67/1
  [cnt_rst] -> acc_cntrl5/3(rst)
  Constant7/1 -> fft_wideband_real3/2(shift)
  simple_bram_vacc45/1(dout) -> Reinterpret49/1
  pipeline272/1(q) -> fft_wideband_real3/1(sync)
  Delay64/1 -> Delay74/1
  munge3/1(dout) -> bus_expand3/1(bus_in)
  power28/1 -> Delay68/1
  simple_bram_vacc43/1(dout) -> Reinterpret51/1
  fft_wideband_real3/5(out03) -> Delay79/1
  adc_snapshot_a/1(arm_out) -> Terminator61/1
  Reinterpret28/1 -> adc_snapshot_a/1(in_d)
  Reinterpret51/1 -> pipeline258/1(d)
  simple_bram_vacc43/2(valid) -> pipeline259/1(d)
  Reinterpret46/1 -> pipeline260/1(d)
  Reinterpret49/1 -> pipeline264/1(d)
  simple_bram_vacc45/2(valid) -> pipeline266/1(d)
  simple_bram_vacc44/2(valid) -> pipeline261/1(d)
  pipeline277/1(q) -> simple_bram_vacc42/1(new_acc)
  pipeline278/1(q) -> simple_bram_vacc42/2(din)
  pipeline166/1(q) -> simple_bram_vacc43/1(new_acc)
  pipeline250/1(q) -> simple_bram_vacc43/2(din)
  pipeline265/1(q) -> simple_bram_vacc44/1(new_acc)
  pipeline270/1(q) -> simple_bram_vacc44/2(din)
  pipeline256/1(q) -> simple_bram_vacc45/1(new_acc)
  pipeline257/1(q) -> simple_bram_vacc45/2(din)
  acc_cntrl5/1(new_acc) -> pipeline263/1(d), pipeline256/1(d), pipeline265/1(d), pipeline166/1(d), pipeline277/1(d)
  pipeline271/1(q) -> pipeline278/1(d)
  pipeline273/1(q) -> pipeline250/1(d)
  pipeline275/1(q) -> pipeline270/1(d)
  pipeline276/1(q) -> pipeline257/1(d)
  Delay68/1 -> pipeline271/1(d)
  Delay69/1 -> pipeline273/1(d)
  Delay76/1 -> pipeline275/1(d)
  Delay81/1 -> pipeline276/1(d)
  Delay107/1 -> pipeline255/1(d)
  Delay88/1 -> pipeline254/1(d)
  Delay73/1 -> pipeline253/1(d)
  Delay72/1 -> pipeline251/1(d)
  pipeline255/1(q) -> pipeline221/1(d)
  pipeline254/1(q) -> pipeline249/1(d)
  pipeline253/1(q) -> pipeline199/1(d)
  pipeline251/1(q) -> pipeline285/1(d)
  acc_cntrl4/1(new_acc) -> pipeline274/1(d), pipeline188/1(d), pipeline244/1(d), pipeline210/1(d), pipeline304/1(d), pipeline282/1(d), pipeline293/1(d), pipeline284/1(d), pipeline167/1(d), pipeline307/1(d), pipeline317/1(d), pipeline309/1(d), pipeline177/1(d)
  pipeline221/1(q) -> simple_bram_vacc41/2(din)
  pipeline210/1(q) -> simple_bram_vacc41/1(new_acc)
  pipeline249/1(q) -> simple_bram_vacc36/2(din)
  pipeline244/1(q) -> simple_bram_vacc36/1(new_acc)
  pipeline199/1(q) -> simple_bram_vacc25/2(din)
  pipeline188/1(q) -> simple_bram_vacc25/1(new_acc)
  pipeline285/1(q) -> simple_bram_vacc24/2(din)
  pipeline274/1(q) -> simple_bram_vacc24/1(new_acc)
  simple_bram_vacc36/2(valid) -> pipeline240/1(d)
  simple_bram_vacc41/2(valid) -> pipeline245/1(d)
  Reinterpret38/1 -> pipeline243/1(d)
  Reinterpret27/1 -> pipeline239/1(d)
  simple_bram_vacc25/2(valid) -> pipeline238/1(d)
  Reinterpret48/1 -> pipeline232/1(d)
  Reinterpret52/1 -> adc_snapshot_b/1(in_d)
  adc_snapshot_b/1(arm_out) -> Terminator56/1
  fft_wideband_real2/5(out03) -> Delay105/1
  simple_bram_vacc25/1(dout) -> Reinterpret48/1
  [acc_len] -> acc_cntrl4/1(acc_len)
  power17/1 -> Delay72/1
  munge2/1(dout) -> bus_expand2/1(bus_in)
  Delay66/1 -> Delay77/1
  pipeline252/1(q) -> fft_wideband_real2/1(sync)
  simple_bram_vacc41/1(dout) -> Reinterpret38/1
  Constant6/1 -> fft_wideband_real2/2(shift)
  [cnt_rst] -> acc_cntrl4/3(rst)
  Delay82/1 -> Delay71/1
  c2_2/1(data_out) -> Terminator40/1
  Delay62/1 -> Delay63/1
  sync_gen2/1(sync_out) -> Delay70/1
  Delay71/1 -> acc_cntrl4/2(sync)
  simple_bram_vacc36/1(dout) -> Reinterpret27/1
  simple_bram_vacc24/2(valid) -> spect_cnt4/2, pipeline247/1(d)
  power25/1 -> Delay73/1
  power26/1 -> Delay88/1
  Delay70/1 -> pipeline252/1(d)
  Delay60/1 -> Delay56/1
  fft_wideband_real2/4(out02) -> Delay66/1
  fft_wideband_real2/3(out01) -> Delay62/1
  Delay105/1 -> Delay106/1
  fft_wideband_real2/1(sync_out) -> Delay60/1
  Delay61/1 -> Delay99/1
  c2_3/1(data_out) -> Terminator51/1
  Reinterpret47/1 -> pipeline246/1(d)
  c2_0/1(data_out) -> Terminator36/1
  simple_bram_vacc24/1(dout) -> Reinterpret47/1
  fft_wideband_real2/6(of) -> Terminator34/1
  rfdc/5(m20_axis_tdata) -> Reinterpret52/1, munge2/1(din)
  Delay56/1 -> bram_mult0_3/1(In1), bram_mult0_2/1(In1), bram_mult0_1/1(In1), bram_mult0_0/1(In1), pipeline237/1(d), Delay82/1
  fft_wideband_real2/2(out00) -> Delay61/1
  power27/1 -> Delay107/1
  c2_1/1(data_out) -> Terminator37/1
  pipeline177/1(q) -> spect_cnt4/1
  Constant4/1 -> adc_snapshot_b/2(we), adc_snapshot_b/3(trig)
  invert_load/1(in_reg) -> pipeline327/1(d)
  Constant8/1 -> invert_load/1(sim_1)
  inverter4/1(dout) -> comp_add18/2(b), cmult8/2(b)
  inverter1/1(dout) -> comp_add19/2(b), cmult9/2(b)
  inverter2/1(dout) -> comp_add16/2(b), cmult10/2(b)
  inverter3/1(dout) -> comp_add17/2(b), cmult11/2(b)
  [acc_len] -> acc_cntrl8/1(acc_len)
  pipeline338/1(q) -> spect_cnt8/1
  comb1/1(data_out) -> Terminator15/1
  power35/1 -> Delay119/1
  simple_bram_vacc48/1(dout) -> Reinterpret55/1
  spect_cnt8/1 -> pipeline329/1(d), pipeline352/1(d), pipeline337/1(d), pipeline344/1(d)
  comb0/1(data_out) -> Terminator12/1
  Reinterpret55/1 -> pipeline342/1(d)
  comb3/1(data_out) -> Terminator63/1
  pipeline343/1(q) -> comb0/3(we)
  pipeline342/1(q) -> comb0/2(data_in)
  power34/1 -> Delay118/1
  power33/1 -> Delay116/1
  simple_bram_vacc48/2(valid) -> pipeline343/1(d), spect_cnt8/2
  simple_bram_vacc50/1(dout) -> Reinterpret53/1
  Delay114/1 -> acc_cntrl8/2(sync)
  comb2/1(data_out) -> Terminator62/1
  Delay117/1 -> Delay114/1
  [cnt_rst] -> acc_cntrl8/3(rst)
  simple_bram_vacc51/1(dout) -> Reinterpret54/1
  power36/1 -> Delay115/1
  simple_bram_vacc49/1(dout) -> Reinterpret56/1
  pipeline329/1(q) -> comb0/1(addr)
  pipeline352/1(q) -> comb1/1(addr)
  pipeline333/1(q) -> comb1/2(data_in)
  pipeline334/1(q) -> comb1/3(we)
  Reinterpret56/1 -> pipeline333/1(d)
  simple_bram_vacc49/2(valid) -> pipeline334/1(d)
  Reinterpret53/1 -> pipeline335/1(d)
  pipeline335/1(q) -> comb2/2(data_in)
  Reinterpret54/1 -> pipeline339/1(d)
  pipeline344/1(q) -> comb3/1(addr)
  pipeline339/1(q) -> comb3/2(data_in)
  pipeline341/1(q) -> comb3/3(we)
  simple_bram_vacc51/2(valid) -> pipeline341/1(d)
  simple_bram_vacc50/2(valid) -> pipeline336/1(d)
  pipeline337/1(q) -> comb2/1(addr)
  pipeline336/1(q) -> comb2/3(we)
  pipeline350/1(q) -> simple_bram_vacc48/1(new_acc)
  pipeline351/1(q) -> simple_bram_vacc48/2(din)
  pipeline328/1(q) -> simple_bram_vacc49/1(new_acc)
  pipeline330/1(q) -> simple_bram_vacc49/2(din)
  pipeline340/1(q) -> simple_bram_vacc50/1(new_acc)
  pipeline345/1(q) -> simple_bram_vacc50/2(din)
  pipeline331/1(q) -> simple_bram_vacc51/1(new_acc)
  pipeline332/1(q) -> simple_bram_vacc51/2(din)
  acc_cntrl8/1(new_acc) -> pipeline338/1(d), pipeline331/1(d), pipeline340/1(d), pipeline328/1(d), pipeline350/1(d)
  pipeline346/1(q) -> pipeline351/1(d)
  pipeline347/1(q) -> pipeline330/1(d)
  pipeline348/1(q) -> pipeline345/1(d)
  pipeline349/1(q) -> pipeline332/1(d)
  Delay115/1 -> pipeline346/1(d)
  Delay116/1 -> pipeline347/1(d)
  Delay118/1 -> pipeline348/1(d)
  Delay119/1 -> pipeline349/1(d)
  comp_add17/1(a+b) -> power35/1
  comp_add16/1(a+b) -> power34/1
  comp_add19/1(a+b) -> power33/1
  comp_add18/1(a+b) -> power36/1
  Delay120/1 -> Delay117/1
  pipeline327/1(q) -> Slice/1
  Slice/1 -> [invert_delay]
  simple_bram_vacc56/1(dout) -> Reinterpret63/1
  Reinterpret61/1 -> pipeline387/1(d)
  pipeline361/1(q) -> simple_bram_vacc53/2(din)
  pipeline376/1(q) -> pipeline373/1(d)
  pipeline386/1(q) -> comb_im2/3(we)
  pipeline357/1(q) -> comb_re2/1(addr)
  Delay123/1 -> pipeline375/1(d)
  c_to_ri11/1 -> Delay127/1
  comb_im2/1(data_out) -> Terminator69/1
  simple_bram_vacc56/2(valid) -> pipeline394/1(d)
  pipeline377/1(q) -> pipeline361/1(d)
  pipeline385/1(q) -> comb_im2/2(data_in)
  Reinterpret62/1 -> pipeline390/1(d)
  pipeline373/1(q) -> simple_bram_vacc52/2(din)
  simple_bram_vacc59/1(dout) -> Reinterpret60/1
  Delay127/1 -> pipeline399/1(d)
  pipeline353/1(q) -> simple_bram_vacc56/1(new_acc)
  simple_bram_vacc52/2(valid) -> pipeline365/1(d)
  pipeline374/1(q) -> pipeline379/1(d)
  simple_bram_vacc57/2(valid) -> pipeline386/1(d)
  pipeline394/1(q) -> comb_re2/3(we)
  pipeline393/1(q) -> comb_re2/2(data_in)
  cmult11/1(ab) -> c_to_ri11/1
  simple_bram_vacc52/1(dout) -> Reinterpret57/1
  comb_re2/1(data_out) -> Terminator68/1
  pipeline381/1(q) -> simple_bram_vacc57/1(new_acc)
  pipeline369/1(q) -> comb_im1/3(we)
  cmult9/1(ab) -> c_to_ri9/1
  comb_re1/1(data_out) -> Terminator66/1
  pipeline392/1(q) -> comb_im3/3(we)
  pipeline358/1(q) -> simple_bram_vacc59/1(new_acc)
  simple_bram_vacc55/1(dout) -> Reinterpret62/1
  simple_bram_vacc54/1(dout) -> Reinterpret61/1
  pipeline368/1(q) -> simple_bram_vacc52/1(new_acc)
  pipeline395/1(q) -> comb_im3/1(addr)
  pipeline398/1(q) -> pipeline382/1(d)
  Reinterpret60/1 -> pipeline362/1(d)
  Delay125/1 -> pipeline376/1(d)
  pipeline356/1(q) -> comb_re0/1(addr)
  Delay124/1 -> pipeline398/1(d)
  simple_bram_vacc59/2(valid) -> pipeline363/1(d)
  pipeline397/1(q) -> pipeline354/1(d)
  pipeline355/1(q) -> comb_im2/1(addr)
  c_to_ri10/2 -> Delay124/1
  Delay122/1 -> pipeline374/1(d)
  cmult8/1(ab) -> c_to_ri8/1
  simple_bram_vacc53/1(dout) -> Reinterpret58/1
  Delay128/1 -> pipeline400/1(d)
  c_to_ri8/1 -> Delay122/1
  comb_im0/1(data_out) -> Terminator65/1
  pipeline389/1(q) -> comb_re3/1(addr)
  pipeline365/1(q) -> comb_re1/3(we)
  pipeline384/1(q) -> simple_bram_vacc55/2(din)
  Reinterpret57/1 -> pipeline364/1(d)
  pipeline378/1(q) -> simple_bram_vacc58/1(new_acc)
  simple_bram_vacc54/2(valid) -> pipeline388/1(d)
  cmult10/1(ab) -> c_to_ri10/1
  simple_bram_vacc58/2(valid) -> spect_cnt9/2, pipeline371/1(d)
  c_to_ri9/2 -> Delay126/1
  pipeline354/1(q) -> simple_bram_vacc56/2(din)
  simple_bram_vacc53/2(valid) -> pipeline369/1(d)
  spect_cnt9/1 -> pipeline357/1(d), pipeline395/1(d), pipeline389/1(d), pipeline355/1(d), pipeline372/1(d), pipeline366/1(d), pipeline380/1(d), pipeline356/1(d)
  pipeline391/1(q) -> simple_bram_vacc54/1(new_acc)
  pipeline372/1(q) -> comb_im1/1(addr)
  pipeline367/1(q) -> comb_im1/2(data_in)
  pipeline382/1(q) -> simple_bram_vacc57/2(din)
  pipeline396/1(q) -> simple_bram_vacc54/2(din)
  Reinterpret58/1 -> pipeline367/1(d)
  comb_re3/1(data_out) -> Terminator70/1
  c_to_ri8/2 -> Delay123/1
  pipeline383/1(q) -> simple_bram_vacc55/1(new_acc)
  pipeline364/1(q) -> comb_re1/2(data_in)
  pipeline360/1(q) -> simple_bram_vacc53/1(new_acc)
  pipeline387/1(q) -> comb_re3/2(data_in)
  simple_bram_vacc58/1(dout) -> Reinterpret59/1
  pipeline399/1(q) -> pipeline396/1(d)
  pipeline363/1(q) -> comb_im0/3(we)
  pipeline359/1(q) -> simple_bram_vacc59/2(din)
  pipeline390/1(q) -> comb_im3/2(data_in)
  comb_im1/1(data_out) -> Terminator67/1
  Reinterpret63/1 -> pipeline393/1(d)
  simple_bram_vacc57/1(dout) -> Reinterpret64/1
  pipeline370/1(q) -> comb_re0/2(data_in)
  pipeline400/1(q) -> pipeline384/1(d)
  pipeline362/1(q) -> comb_im0/2(data_in)
  c_to_ri11/2 -> Delay128/1
  simple_bram_vacc55/2(valid) -> pipeline392/1(d)
  pipeline379/1(q) -> simple_bram_vacc58/2(din)
  pipeline366/1(q) -> comb_re1/1(addr)
  pipeline388/1(q) -> comb_re3/3(we)
  pipeline371/1(q) -> comb_re0/3(we)
  comb_re0/1(data_out) -> Terminator64/1
  Reinterpret59/1 -> pipeline370/1(d)
  c_to_ri10/1 -> Delay121/1
  c_to_ri9/1 -> Delay125/1
  Delay126/1 -> pipeline377/1(d)
  pipeline375/1(q) -> pipeline359/1(d)
  Reinterpret64/1 -> pipeline385/1(d)
  pipeline380/1(q) -> comb_im0/1(addr)
  Delay121/1 -> pipeline397/1(d)
  acc_cntrl9/1(new_acc) -> pipeline383/1(d), pipeline391/1(d), pipeline381/1(d), pipeline353/1(d), pipeline360/1(d), pipeline368/1(d), pipeline358/1(d), pipeline378/1(d), pipeline401/1(d)
  comb_im3/1(data_out) -> Terminator71/1
  Delay109/1 -> comp_add18/1(a), cmult8/1(a)
  Delay110/1 -> comp_add19/1(a), cmult9/1(a)
  Delay111/1 -> comp_add16/1(a), cmult10/1(a)
  Delay112/1 -> comp_add17/1(a), cmult11/1(a)
  [cnt_rst] -> acc_cntrl9/3(rst)
  Delay129/1 -> acc_cntrl9/2(sync)
  pipeline401/1(q) -> spect_cnt9/1
  [acc_len] -> acc_cntrl9/1(acc_len)
  Delay113/1 -> Delay120/1, Delay130/1
  Delay130/1 -> Delay129/1
  [invert_delay] -> inverter1/3(delay)
  [invert_delay] -> inverter2/3(delay)
  [invert_delay] -> inverter3/3(delay)
  [invert_delay] -> inverter4/3(delay)
  pipeline241/1(q) -> a2_0/1(addr)
  pipeline267/1(q) -> a2_0/2(data_in)
  pipeline268/1(q) -> a2_0/3(we)
  pipeline279/1(q) -> a2_1/1(addr)
  pipeline258/1(q) -> a2_1/2(data_in)
  pipeline259/1(q) -> a2_1/3(we)
  pipeline262/1(q) -> a2_2/1(addr)
  pipeline260/1(q) -> a2_2/2(data_in)
  pipeline261/1(q) -> a2_2/3(we)
  pipeline269/1(q) -> a2_3/1(addr)
  pipeline264/1(q) -> a2_3/2(data_in)
  pipeline266/1(q) -> a2_3/3(we)
  pipeline165/1(q) -> b2_0/1(addr)
  pipeline246/1(q) -> b2_0/2(data_in)
  pipeline247/1(q) -> b2_0/3(we)
  pipeline296/1(q) -> b2_1/1(addr)
  pipeline232/1(q) -> b2_1/2(data_in)
  pipeline238/1(q) -> b2_1/3(we)
  pipeline242/1(q) -> b2_2/1(addr)
  pipeline239/1(q) -> b2_2/2(data_in)
  pipeline240/1(q) -> b2_2/3(we)
  pipeline248/1(q) -> b2_3/1(addr)
  pipeline243/1(q) -> b2_3/2(data_in)
  pipeline245/1(q) -> b2_3/3(we)
  pipeline280/1(q) -> ab_re0/1(addr)
  pipeline295/1(q) -> ab_re0/2(data_in)
  pipeline297/1(q) -> ab_re0/3(we)
  pipeline306/1(q) -> ab_im0/1(addr)
  pipeline287/1(q) -> ab_im0/2(data_in)
  pipeline288/1(q) -> ab_im0/3(we)
  pipeline291/1(q) -> ab_re1/1(addr)
  pipeline289/1(q) -> ab_re1/2(data_in)
  pipeline290/1(q) -> ab_re1/3(we)
  pipeline298/1(q) -> ab_im1/1(addr)
  pipeline292/1(q) -> ab_im1/2(data_in)
  pipeline294/1(q) -> ab_im1/3(we)
  pipeline281/1(q) -> ab_re2/1(addr)
  pipeline319/1(q) -> ab_re2/2(data_in)
  pipeline320/1(q) -> ab_re2/3(we)
  pipeline169/1(q) -> ab_im2/1(addr)
  pipeline311/1(q) -> ab_im2/2(data_in)
  pipeline312/1(q) -> ab_im2/3(we)
  pipeline315/1(q) -> ab_re3/1(addr)
  pipeline313/1(q) -> ab_re3/2(data_in)
  pipeline314/1(q) -> ab_re3/3(we)
  pipeline321/1(q) -> ab_im3/1(addr)
  pipeline316/1(q) -> ab_im3/2(data_in)
  pipeline318/1(q) -> ab_im3/3(we)
  pipeline2/1(q) -> c2_0/1(addr)
  pipeline43/1(q) -> c2_0/2(data_in)
  pipeline44/1(q) -> c2_0/3(we)
  pipeline54/1(q) -> c2_1/1(addr)
  pipeline35/1(q) -> c2_1/2(data_in)
  pipeline36/1(q) -> c2_1/3(we)
  pipeline39/1(q) -> c2_2/1(addr)
  pipeline37/1(q) -> c2_2/2(data_in)
  pipeline38/1(q) -> c2_2/3(we)
  pipeline45/1(q) -> c2_3/1(addr)
  pipeline40/1(q) -> c2_3/2(data_in)
  pipeline42/1(q) -> c2_3/3(we)
  pipeline1/1(q) -> d2_0/1(addr)
  pipeline24/1(q) -> d2_0/2(data_in)
  pipeline25/1(q) -> d2_0/3(we)
  pipeline7/1(q) -> d2_1/1(addr)
  pipeline16/1(q) -> d2_1/2(data_in)
  pipeline17/1(q) -> d2_1/3(we)
  pipeline20/1(q) -> d2_2/1(addr)
  pipeline18/1(q) -> d2_2/2(data_in)
  pipeline19/1(q) -> d2_2/3(we)
  pipeline26/1(q) -> d2_3/1(addr)
  pipeline21/1(q) -> d2_3/2(data_in)
  pipeline23/1(q) -> d2_3/3(we)
  pipeline55/1(q) -> cd_re0/1(addr)
  pipeline69/1(q) -> cd_re0/2(data_in)
  pipeline70/1(q) -> cd_re0/3(we)
  pipeline79/1(q) -> cd_im0/1(addr)
  pipeline61/1(q) -> cd_im0/2(data_in)
  pipeline62/1(q) -> cd_im0/3(we)
  pipeline65/1(q) -> cd_re1/1(addr)
  pipeline63/1(q) -> cd_re1/2(data_in)
  pipeline64/1(q) -> cd_re1/3(we)
  pipeline71/1(q) -> cd_im1/1(addr)
  pipeline66/1(q) -> cd_im1/2(data_in)
  pipeline68/1(q) -> cd_im1/3(we)
  pipeline56/1(q) -> cd_re2/1(addr)
  pipeline92/1(q) -> cd_re2/2(data_in)
  pipeline93/1(q) -> cd_re2/3(we)
  pipeline102/1(q) -> cd_im2/1(addr)
  pipeline84/1(q) -> cd_im2/2(data_in)
  pipeline85/1(q) -> cd_im2/3(we)
  pipeline88/1(q) -> cd_re3/1(addr)
  pipeline86/1(q) -> cd_re3/2(data_in)
  pipeline87/1(q) -> cd_re3/3(we)
  pipeline94/1(q) -> cd_im3/1(addr)
  pipeline89/1(q) -> cd_im3/2(data_in)
  pipeline91/1(q) -> cd_im3/3(we)
  pipeline171/1(q) -> synth1_0/1(addr)
  pipeline185/1(q) -> synth1_0/2(data_in)
  pipeline186/1(q) -> synth1_0/3(we)
  pipeline196/1(q) -> synth1_1/1(addr)
  pipeline175/1(q) -> synth1_1/2(data_in)
  pipeline176/1(q) -> synth1_1/3(we)
  pipeline180/1(q) -> synth1_2/1(addr)
  pipeline178/1(q) -> synth1_2/2(data_in)
  pipeline179/1(q) -> synth1_2/3(we)
  pipeline187/1(q) -> synth1_3/1(addr)
  pipeline182/1(q) -> synth1_3/2(data_in)
  pipeline184/1(q) -> synth1_3/3(we)
  pipeline198/1(q) -> synth0_0/1(addr)
  pipeline213/1(q) -> synth0_0/2(data_in)
  pipeline214/1(q) -> synth0_0/3(we)
  pipeline224/1(q) -> synth0_1/1(addr)
  pipeline203/1(q) -> synth0_1/2(data_in)
  pipeline204/1(q) -> synth0_1/3(we)
  pipeline207/1(q) -> synth0_2/1(addr)
  pipeline205/1(q) -> synth0_2/2(data_in)
  pipeline206/1(q) -> synth0_2/3(we)
  pipeline215/1(q) -> synth0_3/1(addr)
  pipeline209/1(q) -> synth0_3/2(data_in)
  pipeline212/1(q) -> synth0_3/3(we)
  pipeline104/1(q) -> synth2_0/1(addr)
  pipeline117/1(q) -> synth2_0/2(data_in)
  pipeline118/1(q) -> synth2_0/3(we)
  pipeline127/1(q) -> synth2_1/1(addr)
  pipeline108/1(q) -> synth2_1/2(data_in)
  pipeline109/1(q) -> synth2_1/3(we)
  pipeline112/1(q) -> synth2_2/1(addr)
  pipeline110/1(q) -> synth2_2/2(data_in)
  pipeline111/1(q) -> synth2_2/3(we)
  pipeline119/1(q) -> synth2_3/1(addr)
  pipeline114/1(q) -> synth2_3/2(data_in)
  pipeline116/1(q) -> synth2_3/3(we)
  pipeline129/1(q) -> synth3_0/1(addr)
  pipeline142/1(q) -> synth3_0/2(data_in)
  pipeline143/1(q) -> synth3_0/3(we)
  pipeline152/1(q) -> synth3_1/1(addr)
  pipeline133/1(q) -> synth3_1/2(data_in)
  pipeline134/1(q) -> synth3_1/3(we)
  pipeline137/1(q) -> synth3_2/1(addr)
  pipeline135/1(q) -> synth3_2/2(data_in)
  pipeline136/1(q) -> synth3_2/3(we)
  pipeline144/1(q) -> synth3_3/1(addr)
  pipeline139/1(q) -> synth3_3/2(data_in)
  pipeline141/1(q) -> synth3_3/3(we)
  Delay59/1 -> power29/1, cmult5/1(a), pipeline230/1(d), bram_mult0_1/2(d)
  Delay74/1 -> power30/1, cmult6/1(a), pipeline231/1(d), bram_mult0_2/2(d)
  Reinterpret76/1 -> Convert4/1
  Reinterpret77/1 -> Convert5/1
  Reinterpret79/1 -> Convert8/1
  Reinterpret78/1 -> Convert6/1
  Reinterpret74/1 -> Convert2/1
  Reinterpret75/1 -> Convert3/1
  Reinterpret73/1 -> Convert1/1
  Reinterpret72/1 -> Convert7/1
  bus_expand/1(msb_out8) -> Reinterpret72/1
  bus_expand/2(out7) -> Reinterpret73/1
  bus_expand/3(out6) -> Reinterpret74/1
  bus_expand/4(out5) -> Reinterpret75/1
  bus_expand/5(out4) -> Reinterpret76/1
  bus_expand/6(out3) -> Reinterpret77/1
  bus_expand/7(out2) -> Reinterpret78/1
  bus_expand/8(lsb_out1) -> Reinterpret79/1
  Convert7/1 -> fft_wideband_real/3(in00)
  Convert1/1 -> fft_wideband_real/4(in01)
  Convert2/1 -> fft_wideband_real/5(in02)
  Convert3/1 -> fft_wideband_real/6(in03)
  Convert4/1 -> fft_wideband_real/7(in04)
  Convert5/1 -> fft_wideband_real/8(in05)
  Convert6/1 -> fft_wideband_real/9(in06)
  Convert8/1 -> fft_wideband_real/10(in07)
  Convert16/1 -> fft_wideband_real1/10(in07)
  Convert14/1 -> fft_wideband_real1/9(in06)
  Convert13/1 -> fft_wideband_real1/8(in05)
  Convert12/1 -> fft_wideband_real1/7(in04)
  Convert11/1 -> fft_wideband_real1/6(in03)
  Convert10/1 -> fft_wideband_real1/5(in02)
  Convert9/1 -> fft_wideband_real1/4(in01)
  Convert15/1 -> fft_wideband_real1/3(in00)
  bus_expand1/8(lsb_out1) -> Reinterpret80/1
  bus_expand1/7(out2) -> Reinterpret71/1
  bus_expand1/6(out3) -> Reinterpret70/1
  bus_expand1/5(out4) -> Reinterpret69/1
  bus_expand1/4(out5) -> Reinterpret68/1
  bus_expand1/3(out6) -> Reinterpret67/1
  bus_expand1/2(out7) -> Reinterpret66/1
  bus_expand1/1(msb_out8) -> Reinterpret65/1
  Reinterpret65/1 -> Convert15/1
  Reinterpret66/1 -> Convert9/1
  Reinterpret68/1 -> Convert11/1
  Reinterpret67/1 -> Convert10/1
  Reinterpret71/1 -> Convert14/1
  Reinterpret80/1 -> Convert16/1
  Reinterpret70/1 -> Convert13/1
  Reinterpret69/1 -> Convert12/1
  Reinterpret85/1 -> Convert19/1
  Reinterpret86/1 -> Convert20/1
  Reinterpret88/1 -> Convert23/1
  Reinterpret87/1 -> Convert21/1
  Reinterpret83/1 -> Convert17/1
  Reinterpret84/1 -> Convert18/1
  Reinterpret82/1 -> Convert24/1
  Reinterpret81/1 -> Convert22/1
  bus_expand2/1(msb_out8) -> Reinterpret81/1
  bus_expand2/2(out7) -> Reinterpret82/1
  bus_expand2/3(out6) -> Reinterpret83/1
  bus_expand2/4(out5) -> Reinterpret84/1
  bus_expand2/5(out4) -> Reinterpret85/1
  bus_expand2/6(out3) -> Reinterpret86/1
  bus_expand2/7(out2) -> Reinterpret87/1
  bus_expand2/8(lsb_out1) -> Reinterpret88/1
  Convert22/1 -> fft_wideband_real2/3(in00)
  Convert24/1 -> fft_wideband_real2/4(in01)
  Convert17/1 -> fft_wideband_real2/5(in02)
  Convert18/1 -> fft_wideband_real2/6(in03)
  Convert19/1 -> fft_wideband_real2/7(in04)
  Convert20/1 -> fft_wideband_real2/8(in05)
  Convert21/1 -> fft_wideband_real2/9(in06)
  Convert23/1 -> fft_wideband_real2/10(in07)
  Convert31/1 -> fft_wideband_real3/10(in07)
  Convert29/1 -> fft_wideband_real3/9(in06)
  Convert28/1 -> fft_wideband_real3/8(in05)
  Convert27/1 -> fft_wideband_real3/7(in04)
  Convert26/1 -> fft_wideband_real3/6(in03)
  Convert25/1 -> fft_wideband_real3/5(in02)
  Convert32/1 -> fft_wideband_real3/4(in01)
  Convert30/1 -> fft_wideband_real3/3(in00)
  bus_expand3/8(lsb_out1) -> Reinterpret96/1
  bus_expand3/7(out2) -> Reinterpret95/1
  bus_expand3/6(out3) -> Reinterpret94/1
  bus_expand3/5(out4) -> Reinterpret93/1
  bus_expand3/4(out5) -> Reinterpret92/1
  bus_expand3/3(out6) -> Reinterpret91/1
  bus_expand3/2(out7) -> Reinterpret90/1
  bus_expand3/1(msb_out8) -> Reinterpret89/1
  Reinterpret89/1 -> Convert30/1
  Reinterpret90/1 -> Convert32/1
  Reinterpret92/1 -> Convert26/1
  Reinterpret91/1 -> Convert25/1
  Reinterpret95/1 -> Convert29/1
  Reinterpret96/1 -> Convert31/1
  Reinterpret94/1 -> Convert28/1
  Reinterpret93/1 -> Convert27/1
